simulink library "ee_lib_for_power_systems"  (R2025b)
settings: Solver=VariableStepAuto  StartTime=0.0  StopTime=10.0  RelTol=1e-3
undefined symbols (not defined in the file or in its folder): Alt_PolePair_Count, C3m, DiscIntNeverNeededParam, Enum1to12, L3m, Total_SM_Inertia, Ts
libraries: fl_lib x291, nesl_utility x219, ee_lib x139, custom_lib x12, $bdroot x10, ee_sl_lib x3, eeTransforms x2, eeGeneralControl x1, simulink x1
(part 1 of 11)
settings Model: Creator=gdudgeon  LastModifiedBy=gdudgeon

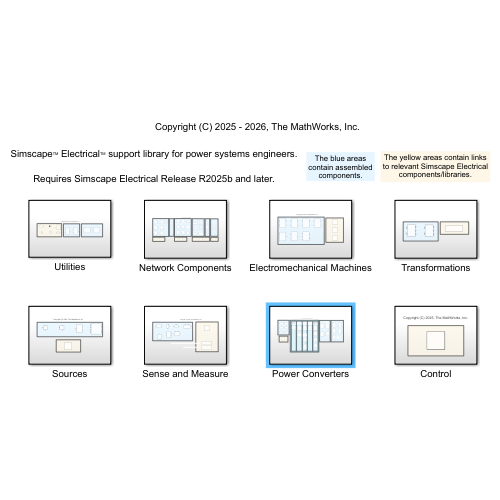
[thumbnail: Simulink's stored preview of the top-level diagram]
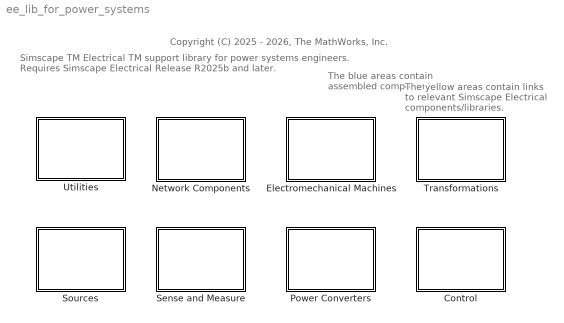
== system ee_lib_for_power_systems ==
  note: The blue areas contain assembled components.
  note: Simscape TM Electrical TM support library for power systems engineers.
      |
      | Requires Simscape Electrical Release R2025b and later.
  note: Copyright (C) 2025 - 2026, The MathWorks, Inc.
  note: The yellow areas contain links to relevant Simscape Electrical components/libraries.
  Control : Subsystem
  Electromechanical Machines : Subsystem
  Network Components : Subsystem
  Power Converters : Subsystem
  Sense and Measure : Subsystem
  Sources : Subsystem
  Transformations : Subsystem
  Utilities : Subsystem

== system ee_lib_for_power_systems/Control ==
  note: Link to Simscape Electrical library
  note: Copyright (C) 2025, The MathWorks, Inc .
  Control : ee_lib/Control  Tag=simscape_sublibrary

== system ee_lib_for_power_systems/Electromechanical Machines ==
  note: Link to Simscape Electrical libraries
  note: Copyright (C) 2025, The MathWorks, Inc.
  Asynchronous : ee_lib/Electromechanical/Asynchronous  Tag=simscape_sublibrary  SystemSampleTime=-1  VariantActivationTime=update diagram  EmptyChoice=off  PassThrough=off  PassThroughVariantControl=(default)  EmptyChoiceVariantControl=(default)  AllowFlexibleInterface=on  Latency=0  AutoFrameSizeCalculation=off
  Induction Machine Squirrel Cage (SI) : Subsystem  mask{run_time=on, Pnom=15e3, Vnom=220, Fnom=60, p=1, Rs=0.25, Xls=0.4, Rrp=0.14, Xlrp=0.41, Xm=17, J=100}
      mask prompts: run_time "Set parameters to run-time (model does not recompile if parameters change value)"; Pnom "Pnom (VA)"; Vnom "Vnom (Vrms)"; Fnom "Fnom (Hz)"; p "Pole Pairs"; Rs "Rs (Ohm)"; Xls "Xls (Ohm)"; Rrp "Rr' (Ohm)"; Xlrp "Xlr' (Ohm)"; Xm "Xm (Ohm)"; J "Inertia (kg*m^2)"
      mask Callback run_time:
      | run_time = get_param(gcb,'run_time');
      | 
      | path_induction_machine = [gcb,'/induction_machine'];
      | path_inertia           = [gcb,'/inertia'];
      | 
      | switch run_time
      |     case 'off'
      |         pcr = 'compiletime';
      |     case 'on'
      |         pcr = 'runtime';
      | end
      | 
      | set_param(path_induction_machine,'SRated_conf',pcr)
      | set_param(path_induction_machine,'VRated_conf',pcr)
      | set_param(path_induction_machine,'FRated_conf',pcr)
      | set_param(path_induction_machine,'nPolePairs_conf',pcr)
      | set_param(path_induction_machine,'Rs_conf',pcr)
      | set_param(path_induction_machine,'Xls_conf',pcr)
      | set_param(path_induction_machine,'Rrd_conf',pcr)
      | set_param(path_induction_machine,'Xlrd_conf',pcr)
      | set_param(path_induction_machine,'Xm_conf',pcr)
      | 
      | set_param(path_inertia,'SRated_conf',pcr)
      | set_param(path_inertia,'FRated_conf',pcr)
      | set_param(path_inertia,'nPolePairs_conf',pcr)
      | set_param(path_inertia,'J_conf',pcr)
      mask DialogControl DescGroupVar Prompt: Induction Machine Squirrel Cage (SI)
      mask Display: disp('Induction\nMachine\nSquirrel\nCage (SI)')
  PMSM (SI) : Subsystem  mask{run_time=on, p=2, flux_linkage=0.5, Ld=0.0002, Lq=0.0002, Rs=0.01, J=0.01, D=0}
      mask prompts: run_time "Set parameters to run-time (model does not recompile if parameters change value)"; p "pole pairs"; flux_linkage "Flux linkage (Wb)"; Ld "Stator d-axis inductance (H)"; Lq "Stator q-axis inductance (H)"; Rs "Stator resistance per phase (Ohm)"; J "Rotor inertia (kg*m^2)"; D "Rotor damping (N*m*s/rad)"
      mask Callback run_time:
      | run_time = get_param(gcb,'run_time');
      | 
      | path_pmsm       = [gcb,'/PMSM'];
      | 
      | switch run_time
      |     case 'off'
      |         pcr = 'compiletime';
      |     case 'on'
      |         pcr = 'runtime';
      | end
      | 
      | set_param(path_pmsm,'nPolePairs_conf',pcr)
      | set_param(path_pmsm,'pm_flux_linkage_conf',pcr)
      | set_param(path_pmsm,'Ld_conf',pcr)
      | set_param(path_pmsm,'Lq_conf',pcr)
      | set_param(path_pmsm,'Rs_conf',pcr)
      | set_param(path_pmsm,'lam_conf',pcr)
      mask DialogControl DescGroupVar Prompt: PMSM (SI)
      mask Display: disp('PMSM\n(SI)')
  Permanent Magnet : ee_lib/Electromechanical/Permanent Magnet  Tag=simscape_sublibrary  SystemSampleTime=-1  VariantActivationTime=update diagram  EmptyChoice=off  PassThrough=off  PassThroughVariantControl=(default)  EmptyChoiceVariantControl=(default)  AllowFlexibleInterface=on  Latency=0  AutoFrameSizeCalculation=off
  Round Rotor Standard (PU) : Subsystem  mask{Pnom=1e6, Vnom=20e3, Fnom=60, Xd=1.5, Xdp=0.4, Xdpp=0.25, Xq=1.4, Xqp=0.5, Xqpp=0.25, Xl=0.1, Ra=0.05, D_Q=[Short-Circuit Short-Circuit], Tdp=1.2, Tdpp=0.05, Tdop=7, Tdopp=0.05, Tqp=1, Tqpp=0.05, Tqop=1, Tqopp=0.04, H=0.2, D=0, p=4}
      mask prompts: Pnom "Nominal Power (VA)"; Vnom "Nominal Voltage (Vrms)"; Fnom "Nominal Frequency (Hz)"; D_Q "Axis Definition [D Q]"
      mask TypeOptions D_Q: [Short-Circuit Short-Circuit] | [Short-Circuit Open-Circuit] | [Open-Circuit Short-Circuit] | [Open-Circuit Open-Circuit]
      mask Callback D_Q:
      | D_Q = get_param(gcb,'D_Q');
      | 
      | mv = {'on';'on';'on';'on';'on';'on';'on';'on';'on';'on';'on';'on';'on';'on';'on';'on';'on';'on';'on';'on';'on';'on';'on'};
      | 
      | path_generator        = [gcb,'/generator'];
      | path_field_circuit    = [gcb,'/field_circuit'];
      | 
      | switch D_Q
      | 
      |     case '[Short-Circuit Short-Circuit]'
      | 
      |         mv([16 17 20 21]-1) = {'off';'off';'off';'off'};
      |         set_param(gcb, 'MaskVisibilities', mv)
      | 
      |         set_param(path_generator,'d_option','ee.enum.circuit.short')
      |         set_param(path_generator,'q_option','ee.enum.circuit.short')
      |         set_param(path_field_circuit,'d_option','ee.enum.circuit.short')
      | 
      |     case '[Short-Circuit Open-Circuit]'
      | 
      |         mv([16 17 18 19]-1) = {'off';'off';'off';'off'};
      |         set_param(gcb, 'MaskVisibilities', mv)
      | 
      |         set_param(path_generator,'d_option','ee.enum.circuit.short')
      |         set_param(path_generator,'q_option','ee.enum.circuit.open')
      |         set_param(path_field_circuit,'d_option','ee.enum.circuit.short')
      | 
      |     case '[Open-Circuit Short-Circuit]'
      | 
      |         mv([14 15 20 21]-1) = {'off';'off';'off';'off'};
      |         set_param(gcb, 'MaskVisibilities', mv)
      | 
      |         set_param(path_generator,'d_option','ee.enum.circuit.open')
      |         set_param(path_generator,'q_option','ee.enum.circuit.short')
      |         set_param(path_field_circuit,'d_option','ee.enum.circuit.open')
      | 
      |     case '[Open-Circuit Open-Circuit]'
      | 
      |         mv([14 15 18 19]-1) = {'off';'off';'off';'off'};
      |         set_param(gcb, 'MaskVisibilities', mv)
      | 
      |         set_param(path_generator,'d_option','ee.enum.circuit.open')
      |         set_param(path_generator,'q_option','ee.enum.circuit.open')
      |         set_param(path_field_circuit,'d_option','ee.enum.circuit.open')
      | 
      | end
      mask DialogControl DescGroupVar Prompt: Round Rotor Standard (PU)
      mask DialogControl DescTextVar Prompt: Synchronous machine with a round rotor parameterized using standard per-unit parameters.
      mask DialogControl Container4 Prompt: Reactances
      mask DialogControl Container5 Prompt: Time Constants
      mask DialogControl Container6 Prompt: Mechanical
      mask Display: disp('Round\nRotor (PU)')
  Salient Pole Fundamental (SI) : Subsystem  mask{Pnom=2.5e6, Vnom=460, Fnom=60, Ifd=1000, Ladu_si=0.0005276, Laq_si=0.0003862, Ll_si=1.123e-5, Ra_si=0.00076, Lfd_si=0.0001147, Rfd_si=0.0001743, L1d_si=0.0008393, R1d_si=0.02392, L1q_si=5.371e-5, R1q_si=0.002154, J=100, F=0.65, p=2}
      mask prompts: Pnom "Nominal Power (VA)"; Vnom "Nominal Voltage (Vrms)"; Fnom "Nominal Frequency (Hz)"; Ifd "Field Current (A)"; Ladu_si "Ladu (H)"; Laq_si "Laq (H)"; Ll_si "Ll (H)"; Ra_si "Ra (Ohm)"; Lfd_si "Lfd (H)"; Rfd_si "Rfd (Ohm)"; L1d_si "L1d (H)"; R1d_si "R1d (Ohm)"; L1q_si "L1q (H)"; R1q_si "R1q (Ohm)"; J "J (kg*m^2)"; F "F (N*m*s/rad)"; p "Pole pairs"
      mask init:
      | maskObj = Simulink.Mask.get(gcbh);
      | workspaceObj = maskObj.getWorkspace();
      | 
      | Pnom = workspaceObj.get('Pnom');
      | Vnom = workspaceObj.get('Vnom');
      | Fnom = workspaceObj.get('Fnom');
      | 
      | Ladu_si = workspaceObj.get('Ladu_si');
      | Laq_si  = workspaceObj.get('Laq_si');
      | Ll_si   = workspaceObj.get('Ll_si');
      | Ra_si   = workspaceObj.get('Ra_si');
      | Lfd_si  = workspaceObj.get('Lfd_si');
      | Rfd_si  = workspaceObj.get('Rfd_si');
      | L1d_si  = workspaceObj.get('L1d_si');
      | R1d_si  = workspaceObj.get('R1d_si');
      | L1q_si  = workspaceObj.get('L1q_si');
      | R1q_si  = workspaceObj.get('R1q_si');
      | 
      | Rbase = Vnom^2/Pnom;
      | Lbase = Rbase/(2*pi*Fnom);
      | 
      | Ladu_pu = Ladu_si/Lbase;
      | Laq_pu  = Laq_si/Lbase;
      | Ll_pu   = Ll_si/Lbase;
      | Ra_pu   = Ra_si/Rbase;
      | Lfd_pu  = Lfd_si/Lbase;
      | Rfd_pu  = Rfd_si/Rbase;
      | L1d_pu  = L1d_si/Lbase;
      | R1d_pu  = R1d_si/Rbase;
      | L1q_pu  = L1q_si/Lbase;
      | R1q_pu  = R1q_si/Rbase;
      mask DialogControl DescGroupVar Prompt: Salient Pole Generator
      mask DialogControl Container4 Prompt: Impedances
      mask DialogControl Container6 Prompt: Mechanical
      mask Display: disp('Salient Pole\nFund (SI)')
  Salient Pole Standard (PU) : Subsystem  mask{Pnom=1e6, Vnom=20e3, Fnom=60, Xd=1.5, Xdp=0.4, Xdpp=0.25, Xq=1.4, Xqpp=0.25, Xl=0.1, Ra=0.05, D_Q=[Open-Circuit Open-Circuit], Tdp=1.2, Tdpp=0.05, Tdop=7, Tdopp=0.05, Tqpp=0.05, Tqopp=0.04, H=0.2, D=0, p=4}
      mask prompts: Pnom "Nominal Power (VA)"; Vnom "Nominal Voltage (Vrms)"; Fnom "Nominal Frequency (Hz)"; D_Q "Axis Definition [D Q]"
      mask TypeOptions D_Q: [Short-Circuit Short-Circuit] | [Short-Circuit Open-Circuit] | [Open-Circuit Short-Circuit] | [Open-Circuit Open-Circuit]
      mask Callback D_Q:
      | D_Q = get_param(gcb,'D_Q');
      | 
      | mv = {'on';'on';'on';'on';'on';'on';'on';'on';'on';'on';'on';'on';'on';'on';'on';'on';'on';'on';'on';'on'};
      | 
      | path_generator        = [gcb,'/generator'];
      | path_field_circuit    = [gcb,'/field_circuit'];
      | 
      | switch D_Q
      | 
      |     case '[Short-Circuit Short-Circuit]'
      | 
      |         mv([15 16 18]-1) = {'off';'off';'off'};
      |         set_param(gcb, 'MaskVisibilities', mv)
      | 
      |         set_param(path_generator,'d_option','ee.enum.circuit.short')
      |         set_param(path_generator,'q_option','ee.enum.circuit.short')
      |         set_param(path_field_circuit,'d_option','ee.enum.circuit.short')
      | 
      |     case '[Short-Circuit Open-Circuit]'
      | 
      |         mv([15 16 17]-1) = {'off';'off';'off'};
      |         set_param(gcb, 'MaskVisibilities', mv)
      | 
      |         set_param(path_generator,'d_option','ee.enum.circuit.short')
      |         set_param(path_generator,'q_option','ee.enum.circuit.open')
      |         set_param(path_field_circuit,'d_option','ee.enum.circuit.short')
      | 
      |     case '[Open-Circuit Short-Circuit]'
      | 
      |         mv([13 14 18]-1) = {'off';'off';'off'};
      |         set_param(gcb, 'MaskVisibilities', mv)
      | 
      |         set_param(path_generator,'d_option','ee.enum.circuit.open')
      |         set_param(path_generator,'q_option','ee.enum.circuit.short')
      |         set_param(path_field_circuit,'d_option','ee.enum.circuit.open')
      | 
      |     case '[Open-Circuit Open-Circuit]'
      | 
      |         mv([13 14 17]-1) = {'off';'off';'off'};
      |         set_param(gcb, 'MaskVisibilities', mv)
      | 
      |         set_param(path_generator,'d_option','ee.enum.circuit.open')
      |         set_param(path_generator,'q_option','ee.enum.circuit.open')
      |         set_param(path_field_circuit,'d_option','ee.enum.circuit.open')
      | 
      | end
      mask DialogControl DescGroupVar Prompt: Salient Pole Generator
      mask DialogControl Container4 Prompt: Reactances
      mask DialogControl Container5 Prompt: Time Constants
      mask DialogControl Container6 Prompt: Mechanical
      mask Display: disp('Salient\nPole (PU)')
  Salient Pole Standard (SI) : Subsystem  mask{Pnom=2.5e6, Vnom=460, Fnom=60, Ladu=0.0005276, Laq=0.0003862, Ll=1.123e-5, Ra=0.00076, Lfd=0.0001147, Rfd=0.0001743, L1d=0.0008393, R1d=0.02392, L1q=5.371e-5, R1q=0.002154, H=Total_SM_Inertia, D=0.65, p=Alt_PolePair_Count}
      mask prompts: Pnom "Nominal Power (VA)"; Vnom "Nominal Voltage (Vrms)"; Fnom "Nominal Frequency (Hz)"; H "J"; D "F"
      mask DialogControl DescGroupVar Prompt: Salient Pole Generator
      mask DialogControl Container4 Prompt: Impedances
      mask DialogControl Container6 Prompt: Mechanical
      mask Display: disp('Salient\nPole (SI)')
  Synchronous : ee_lib/Electromechanical/Synchronous  Tag=simscape_sublibrary  SystemSampleTime=-1  VariantActivationTime=update diagram  EmptyChoice=off  PassThrough=off  PassThroughVariantControl=(default)  EmptyChoiceVariantControl=(default)  AllowFlexibleInterface=on  Latency=0  AutoFrameSizeCalculation=off
  Vectorized Simulink Synchronous Machine (PU) : Subsystem  mask{Pnom=[1e6 1e6 1e6 1e6], Vnom=[135e3 135e3 135e3 135e3], fnom=[60 60 60 60], NPP=[4 4 4 4], Ra=0.05*ones(1,4), Xl=0.1*ones(1,4), Xd=1.5*ones(1,4), Xq=1.4*ones(1,4), Xdp=0.4*ones(1,4), Xqp=0.5*ones(1,4), Xdpp=0.25*ones(1,4), Xqpp=0.25*ones(1,4), Tdop=5*ones(1,4), Tqop=1.5*ones(1,4), Tdopp=0.6*ones(1,4), Tqopp=0.06*ones(1,4), H=4*ones(1,4), D=zeros(1,4), SatX=[0, .8, 1.26, 2.29, 2.97], xVec=[0, .8, 1.08, 1.31, 1.4], no_gens=4}
      mask prompts: Pnom "Nominal power (VA):"; Vnom "Nominal line-to-line voltage (Vrms):"; fnom "Nominal frequency (Hz):"; NPP "Number of pole pairs:"; Ra "Stator resistance Ra:"; Xl "Stator leakage reactance Xl:"; Xd "d-axis reactance Xd:"; Xq "q-axis reactance Xq:"; Xdp "d-axis transient reactance Xd':"; Xqp "q-axis transient reactance Xq':"; Xdpp "d-axis subtransient reactance Xd'':"; Xqpp "q-axis subtransient reactance Xq'':"; Tdop "d-axis transient open-circuit time constant Tdo' (s):"; Tqop "q-axis transient open-circuit time constant Tqo' (s):"; Tdopp "d-axis subtransient open-circuit time constant Tdo''(s):"; Tqopp "q-axis subtransient open-circuit time constant Tqo''(s):"; H "Inertia constant H (s):"; D "damping coefficient D (pu):"; SatX "Field current (pu)"; xVec "Terminal voltage (pu)"; no_gens "Number of generators"
      mask init:
      | % base values
      | Pbase = Pnom;                 % base power
      | Vbase = Vnom./sqrt(3).*sqrt(2); % base voltage, line-neutral, peak value
      | Ibase = Pbase./(1.5.*Vbase);    % base curretn, peak value
      | wbase = 2*pi.*fnom;            % base radial frequency
      mask DialogControl DescGroupVar Prompt: Vectorized Synchronous Machine Round Rotor (PU)
      mask DialogControl DescTextVar Prompt: Implement Round Rotor model for synchronous machine compatible with vectorization. Note that currently only a single saturation curve is supported.
      mask DialogControl Container4 Prompt: Main
      mask DialogControl Container7 Prompt: Impedances
      mask DialogControl Container8 Prompt: Time constants
      mask DialogControl Container12 Prompt: Mechanical
      mask DialogControl Container9 Prompt: Saturation
      mask DialogControl Container11 Prompt: Other
